simulink model "All_64_Coils"  (R2021b) (continued)
== system All_64_Coils/Coil-29 ==
  x : Inport
  y : Inport  Port=2
  z : Inport  Port=3
  Current : Inport  Port=4
  Add{,1..4} (5 alike) : Sum
  Display{,1..3} (4 alike) : Display
  Inner_5_coils : Subsystem
  Outer_5_Coils : Subsystem
  Scope : Scope  NumInputPorts=4
  Scope1 : Scope
  wires:
  Outer_5_Coils/3(Output2) -> Add2/2
  Inner_5_coils/2(Output1) -> Add1/1
  Outer_5_Coils/4(Output3) -> Add3/2
  z/1 -> Outer_5_Coils/3(Input2), Inner_5_coils/3(Input2)
  Add/1 -> Scope/1, Display/1  "Fxx"
  y/1 -> Outer_5_Coils/2(Input1), Inner_5_coils/2(Input1)
  Inner_5_coils/4(Output3) -> Add3/1
  Inner_5_coils/1(Output) -> Add/1
  Add3/1 -> Scope/4, Display3/1, Add4/2  "Fyz"
  Add1/1 -> Scope/2, Display1/1, Add4/1  "Fxz"
  Outer_5_Coils/1(Output) -> Add/2
  x/1 -> Outer_5_Coils/1(Input), Inner_5_coils/1(Input)
  Add2/1 -> Scope/3, Display2/1  "Fyy"
  Outer_5_Coils/2(Output1) -> Add1/2
  Current/1 -> Outer_5_Coils/4(Input3), Inner_5_coils/4(Input3)
  Inner_5_coils/3(Output2) -> Add2/1
  Add4/1 -> Scope1/1  "Total_Force"

== system All_64_Coils/Coil-3 ==
  x : Inport
  y : Inport  Port=2
  z : Inport  Port=3
  Current : Inport  Port=4
  Add{,1..4} (5 alike) : Sum
  Display{,1..3} (4 alike) : Display
  Inner_5_coils : Subsystem
  Outer_5_Coils : Subsystem
  Scope : Scope  NumInputPorts=4
  Scope1 : Scope
  wires:
  Outer_5_Coils/3(Output2) -> Add2/2
  Inner_5_coils/2(Output1) -> Add1/1
  Outer_5_Coils/4(Output3) -> Add3/2
  z/1 -> Outer_5_Coils/3(Input2), Inner_5_coils/3(Input2)
  Add/1 -> Scope/1, Display/1  "Fxx"
  y/1 -> Outer_5_Coils/2(Input1), Inner_5_coils/2(Input1)
  Inner_5_coils/4(Output3) -> Add3/1
  Inner_5_coils/1(Output) -> Add/1
  Add3/1 -> Scope/4, Display3/1, Add4/2  "Fyz"
  Add1/1 -> Scope/2, Display1/1, Add4/1  "Fxz"
  Outer_5_Coils/1(Output) -> Add/2
  x/1 -> Outer_5_Coils/1(Input), Inner_5_coils/1(Input)
  Add2/1 -> Scope/3, Display2/1  "Fyy"
  Outer_5_Coils/2(Output1) -> Add1/2
  Current/1 -> Outer_5_Coils/4(Input3), Inner_5_coils/4(Input3)
  Inner_5_coils/3(Output2) -> Add2/1
  Add4/1 -> Scope1/1  "Total_Force"

== system All_64_Coils/Coil-30 ==
  x : Inport
  y : Inport  Port=2
  z : Inport  Port=3
  Current : Inport  Port=4
  Add{,1..4} (5 alike) : Sum
  Display{,1..3} (4 alike) : Display
  Inner_5_coils : Subsystem
  Outer_5_Coils : Subsystem
  Scope : Scope  NumInputPorts=4
  Scope1 : Scope
  wires:
  Outer_5_Coils/3(Output2) -> Add2/2
  Inner_5_coils/2(Output1) -> Add1/1
  Outer_5_Coils/4(Output3) -> Add3/2
  z/1 -> Outer_5_Coils/3(Input2), Inner_5_coils/3(Input2)
  Add/1 -> Scope/1, Display/1  "Fxx"
  y/1 -> Outer_5_Coils/2(Input1), Inner_5_coils/2(Input1)
  Inner_5_coils/4(Output3) -> Add3/1
  Inner_5_coils/1(Output) -> Add/1
  Add3/1 -> Scope/4, Display3/1, Add4/2  "Fyz"
  Add1/1 -> Scope/2, Display1/1, Add4/1  "Fxz"
  Outer_5_Coils/1(Output) -> Add/2
  x/1 -> Outer_5_Coils/1(Input), Inner_5_coils/1(Input)
  Add2/1 -> Scope/3, Display2/1  "Fyy"
  Outer_5_Coils/2(Output1) -> Add1/2
  Current/1 -> Outer_5_Coils/4(Input3), Inner_5_coils/4(Input3)
  Inner_5_coils/3(Output2) -> Add2/1
  Add4/1 -> Scope1/1  "Total_Force"

== system All_64_Coils/Coil-31 ==
  x : Inport
  y : Inport  Port=2
  z : Inport  Port=3
  Current : Inport  Port=4
  Add{,1..4} (5 alike) : Sum
  Display{,1..3} (4 alike) : Display
  Inner_5_coils : Subsystem
  Outer_5_Coils : Subsystem
  Scope : Scope  NumInputPorts=4
  Scope1 : Scope
  wires:
  Add4/1 -> Scope1/1  "Total_Force"
  Inner_5_coils/3(Output2) -> Add2/1
  Current/1 -> Inner_5_coils/4(Input3), Outer_5_Coils/4(Input3)
  Outer_5_Coils/2(Output1) -> Add1/2
  Add2/1 -> Display2/1, Scope/3  "Fyy"
  x/1 -> Inner_5_coils/1(Input), Outer_5_Coils/1(Input)
  Outer_5_Coils/1(Output) -> Add/2
  Add1/1 -> Add4/1, Display1/1, Scope/2  "Fxz"
  Add3/1 -> Add4/2, Display3/1, Scope/4  "Fyz"
  Inner_5_coils/1(Output) -> Add/1
  Inner_5_coils/4(Output3) -> Add3/1
  y/1 -> Inner_5_coils/2(Input1), Outer_5_Coils/2(Input1)
  Add/1 -> Display/1, Scope/1  "Fxx"
  z/1 -> Inner_5_coils/3(Input2), Outer_5_Coils/3(Input2)
  Outer_5_Coils/4(Output3) -> Add3/2
  Inner_5_coils/2(Output1) -> Add1/1
  Outer_5_Coils/3(Output2) -> Add2/2

== system All_64_Coils/Coil-32 ==
  x : Inport
  y : Inport  Port=2
  z : Inport  Port=3
  Current : Inport  Port=4
  Add{,1..4} (5 alike) : Sum
  Display{,1..3} (4 alike) : Display
  Inner_5_coils : Subsystem
  Outer_5_Coils : Subsystem
  Scope : Scope  NumInputPorts=4
  Scope1 : Scope
  wires:
  Add4/1 -> Scope1/1  "Total_Force"
  Inner_5_coils/3(Output2) -> Add2/1
  Current/1 -> Inner_5_coils/4(Input3), Outer_5_Coils/4(Input3)
  Outer_5_Coils/2(Output1) -> Add1/2
  Add2/1 -> Display2/1, Scope/3  "Fyy"
  x/1 -> Inner_5_coils/1(Input), Outer_5_Coils/1(Input)
  Outer_5_Coils/1(Output) -> Add/2
  Add1/1 -> Add4/1, Display1/1, Scope/2  "Fxz"
  Add3/1 -> Add4/2, Display3/1, Scope/4  "Fyz"
  Inner_5_coils/1(Output) -> Add/1
  Inner_5_coils/4(Output3) -> Add3/1
  y/1 -> Inner_5_coils/2(Input1), Outer_5_Coils/2(Input1)
  Add/1 -> Display/1, Scope/1  "Fxx"
  z/1 -> Inner_5_coils/3(Input2), Outer_5_Coils/3(Input2)
  Outer_5_Coils/4(Output3) -> Add3/2
  Inner_5_coils/2(Output1) -> Add1/1
  Outer_5_Coils/3(Output2) -> Add2/2

== system All_64_Coils/Coil-33 ==
  x : Inport
  y : Inport  Port=2
  z : Inport  Port=3
  Current : Inport  Port=4
  Add{,1..4} (5 alike) : Sum
  Display{,1..3} (4 alike) : Display
  Inner_5_coils : Subsystem
  Outer_5_Coils : Subsystem
  Scope : Scope  NumInputPorts=4
  Scope1 : Scope
  wires:
  Outer_5_Coils/3(Output2) -> Add2/2
  Inner_5_coils/2(Output1) -> Add1/1
  Outer_5_Coils/4(Output3) -> Add3/2
  z/1 -> Outer_5_Coils/3(Input2), Inner_5_coils/3(Input2)
  Add/1 -> Scope/1, Display/1  "Fxx"
  y/1 -> Outer_5_Coils/2(Input1), Inner_5_coils/2(Input1)
  Inner_5_coils/4(Output3) -> Add3/1
  Inner_5_coils/1(Output) -> Add/1
  Add3/1 -> Scope/4, Display3/1, Add4/2  "Fyz"
  Add1/1 -> Scope/2, Display1/1, Add4/1  "Fxz"
  Outer_5_Coils/1(Output) -> Add/2
  x/1 -> Outer_5_Coils/1(Input), Inner_5_coils/1(Input)
  Add2/1 -> Scope/3, Display2/1  "Fyy"
  Outer_5_Coils/2(Output1) -> Add1/2
  Current/1 -> Outer_5_Coils/4(Input3), Inner_5_coils/4(Input3)
  Inner_5_coils/3(Output2) -> Add2/1
  Add4/1 -> Scope1/1  "Total_Force"

== system All_64_Coils/Coil-34 ==
  x : Inport
  y : Inport  Port=2
  z : Inport  Port=3
  Current : Inport  Port=4
  Add{,1..4} (5 alike) : Sum
  Display{,1..3} (4 alike) : Display
  Inner_5_coils : Subsystem
  Outer_5_Coils : Subsystem
  Scope : Scope  NumInputPorts=4
  Scope1 : Scope
  wires:
  Outer_5_Coils/3(Output2) -> Add2/2
  Inner_5_coils/2(Output1) -> Add1/1
  Outer_5_Coils/4(Output3) -> Add3/2
  z/1 -> Outer_5_Coils/3(Input2), Inner_5_coils/3(Input2)
  Add/1 -> Scope/1, Display/1  "Fxx"
  y/1 -> Outer_5_Coils/2(Input1), Inner_5_coils/2(Input1)
  Inner_5_coils/4(Output3) -> Add3/1
  Inner_5_coils/1(Output) -> Add/1
  Add3/1 -> Scope/4, Display3/1, Add4/2  "Fyz"
  Add1/1 -> Scope/2, Display1/1, Add4/1  "Fxz"
  Outer_5_Coils/1(Output) -> Add/2
  x/1 -> Outer_5_Coils/1(Input), Inner_5_coils/1(Input)
  Add2/1 -> Scope/3, Display2/1  "Fyy"
  Outer_5_Coils/2(Output1) -> Add1/2
  Current/1 -> Outer_5_Coils/4(Input3), Inner_5_coils/4(Input3)
  Inner_5_coils/3(Output2) -> Add2/1
  Add4/1 -> Scope1/1  "Total_Force"

== system All_64_Coils/Coil-35 ==
  x : Inport
  y : Inport  Port=2
  z : Inport  Port=3
  Current : Inport  Port=4
  Add{,1..4} (5 alike) : Sum
  Display{,1..3} (4 alike) : Display
  Inner_5_coils : Subsystem
  Outer_5_Coils : Subsystem
  Scope : Scope  NumInputPorts=4
  Scope1 : Scope
  wires:
  Outer_5_Coils/3(Output2) -> Add2/2
  Inner_5_coils/2(Output1) -> Add1/1
  Outer_5_Coils/4(Output3) -> Add3/2
  z/1 -> Outer_5_Coils/3(Input2), Inner_5_coils/3(Input2)
  Add/1 -> Scope/1, Display/1  "Fxx"
  y/1 -> Outer_5_Coils/2(Input1), Inner_5_coils/2(Input1)
  Inner_5_coils/4(Output3) -> Add3/1
  Inner_5_coils/1(Output) -> Add/1
  Add3/1 -> Scope/4, Display3/1, Add4/2  "Fyz"
  Add1/1 -> Scope/2, Display1/1, Add4/1  "Fxz"
  Outer_5_Coils/1(Output) -> Add/2
  x/1 -> Outer_5_Coils/1(Input), Inner_5_coils/1(Input)
  Add2/1 -> Scope/3, Display2/1  "Fyy"
  Outer_5_Coils/2(Output1) -> Add1/2
  Current/1 -> Outer_5_Coils/4(Input3), Inner_5_coils/4(Input3)
  Inner_5_coils/3(Output2) -> Add2/1
  Add4/1 -> Scope1/1  "Total_Force"

== system All_64_Coils/Coil-36 ==
  x : Inport
  y : Inport  Port=2
  z : Inport  Port=3
  Current : Inport  Port=4
  Add{,1..4} (5 alike) : Sum
  Display{,1..3} (4 alike) : Display
  Inner_5_coils : Subsystem
  Outer_5_Coils : Subsystem
  Scope : Scope  NumInputPorts=4
  Scope1 : Scope
  wires:
  Add4/1 -> Scope1/1  "Total_Force"
  Inner_5_coils/3(Output2) -> Add2/1
  Current/1 -> Inner_5_coils/4(Input3), Outer_5_Coils/4(Input3)
  Outer_5_Coils/2(Output1) -> Add1/2
  Add2/1 -> Display2/1, Scope/3  "Fyy"
  x/1 -> Inner_5_coils/1(Input), Outer_5_Coils/1(Input)
  Outer_5_Coils/1(Output) -> Add/2
  Add1/1 -> Add4/1, Display1/1, Scope/2  "Fxz"
  Add3/1 -> Add4/2, Display3/1, Scope/4  "Fyz"
  Inner_5_coils/1(Output) -> Add/1
  Inner_5_coils/4(Output3) -> Add3/1
  y/1 -> Inner_5_coils/2(Input1), Outer_5_Coils/2(Input1)
  Add/1 -> Display/1, Scope/1  "Fxx"
  z/1 -> Inner_5_coils/3(Input2), Outer_5_Coils/3(Input2)
  Outer_5_Coils/4(Output3) -> Add3/2
  Inner_5_coils/2(Output1) -> Add1/1
  Outer_5_Coils/3(Output2) -> Add2/2

== system All_64_Coils/Coil-37 ==
  x : Inport
  y : Inport  Port=2
  z : Inport  Port=3
  Current : Inport  Port=4
  Add{,1..4} (5 alike) : Sum
  Display{,1..3} (4 alike) : Display
  Inner_5_coils : Subsystem
  Outer_5_Coils : Subsystem
  Scope : Scope  NumInputPorts=4
  Scope1 : Scope
  wires:
  Outer_5_Coils/3(Output2) -> Add2/2
  Inner_5_coils/2(Output1) -> Add1/1
  Outer_5_Coils/4(Output3) -> Add3/2
  z/1 -> Outer_5_Coils/3(Input2), Inner_5_coils/3(Input2)
  Add/1 -> Scope/1, Display/1  "Fxx"
  y/1 -> Outer_5_Coils/2(Input1), Inner_5_coils/2(Input1)
  Inner_5_coils/4(Output3) -> Add3/1
  Inner_5_coils/1(Output) -> Add/1
  Add3/1 -> Scope/4, Display3/1, Add4/2  "Fyz"
  Add1/1 -> Scope/2, Display1/1, Add4/1  "Fxz"
  Outer_5_Coils/1(Output) -> Add/2
  x/1 -> Outer_5_Coils/1(Input), Inner_5_coils/1(Input)
  Add2/1 -> Scope/3, Display2/1  "Fyy"
  Outer_5_Coils/2(Output1) -> Add1/2
  Current/1 -> Outer_5_Coils/4(Input3), Inner_5_coils/4(Input3)
  Inner_5_coils/3(Output2) -> Add2/1
  Add4/1 -> Scope1/1  "Total_Force"

== system All_64_Coils/Coil-38 ==
  x : Inport
  y : Inport  Port=2
  z : Inport  Port=3
  Current : Inport  Port=4
  Add{,1..4} (5 alike) : Sum
  Display{,1..3} (4 alike) : Display
  Inner_5_coils : Subsystem
  Outer_5_Coils : Subsystem
  Scope : Scope  NumInputPorts=4
  Scope1 : Scope
  wires:
  Add4/1 -> Scope1/1  "Total_Force"
  Inner_5_coils/3(Output2) -> Add2/1
  Current/1 -> Inner_5_coils/4(Input3), Outer_5_Coils/4(Input3)
  Outer_5_Coils/2(Output1) -> Add1/2
  Add2/1 -> Display2/1, Scope/3  "Fyy"
  x/1 -> Inner_5_coils/1(Input), Outer_5_Coils/1(Input)
  Outer_5_Coils/1(Output) -> Add/2
  Add1/1 -> Add4/1, Display1/1, Scope/2  "Fxz"
  Add3/1 -> Add4/2, Display3/1, Scope/4  "Fyz"
  Inner_5_coils/1(Output) -> Add/1
  Inner_5_coils/4(Output3) -> Add3/1
  y/1 -> Inner_5_coils/2(Input1), Outer_5_Coils/2(Input1)
  Add/1 -> Display/1, Scope/1  "Fxx"
  z/1 -> Inner_5_coils/3(Input2), Outer_5_Coils/3(Input2)
  Outer_5_Coils/4(Output3) -> Add3/2
  Inner_5_coils/2(Output1) -> Add1/1
  Outer_5_Coils/3(Output2) -> Add2/2

== system All_64_Coils/Coil-39 ==
  x : Inport
  y : Inport  Port=2
  z : Inport  Port=3
  Current : Inport  Port=4
  Add{,1..4} (5 alike) : Sum
  Display{,1..3} (4 alike) : Display
  Inner_5_coils : Subsystem
  Outer_5_Coils : Subsystem
  Scope : Scope  NumInputPorts=4
  Scope1 : Scope
  wires:
  Add4/1 -> Scope1/1  "Total_Force"
  Inner_5_coils/3(Output2) -> Add2/1
  Current/1 -> Inner_5_coils/4(Input3), Outer_5_Coils/4(Input3)
  Outer_5_Coils/2(Output1) -> Add1/2
  Add2/1 -> Display2/1, Scope/3  "Fyy"
  x/1 -> Inner_5_coils/1(Input), Outer_5_Coils/1(Input)
  Outer_5_Coils/1(Output) -> Add/2
  Add1/1 -> Add4/1, Display1/1, Scope/2  "Fxz"
  Add3/1 -> Add4/2, Display3/1, Scope/4  "Fyz"
  Inner_5_coils/1(Output) -> Add/1
  Inner_5_coils/4(Output3) -> Add3/1
  y/1 -> Inner_5_coils/2(Input1), Outer_5_Coils/2(Input1)
  Add/1 -> Display/1, Scope/1  "Fxx"
  z/1 -> Inner_5_coils/3(Input2), Outer_5_Coils/3(Input2)
  Outer_5_Coils/4(Output3) -> Add3/2
  Inner_5_coils/2(Output1) -> Add1/1
  Outer_5_Coils/3(Output2) -> Add2/2

== system All_64_Coils/Coil-4 ==
  x : Inport
  y : Inport  Port=2
  z : Inport  Port=3
  Current : Inport  Port=4
  Add{,1..4} (5 alike) : Sum
  Display{,1..3} (4 alike) : Display
  Inner_5_coils : Subsystem
  Outer_5_Coils : Subsystem
  Scope : Scope  NumInputPorts=4
  Scope1 : Scope
  wires:
  Add4/1 -> Scope1/1  "Total_Force"
  Inner_5_coils/3(Output2) -> Add2/1
  Current/1 -> Inner_5_coils/4(Input3), Outer_5_Coils/4(Input3)
  Outer_5_Coils/2(Output1) -> Add1/2
  Add2/1 -> Display2/1, Scope/3  "Fyy"
  x/1 -> Inner_5_coils/1(Input), Outer_5_Coils/1(Input)
  Outer_5_Coils/1(Output) -> Add/2
  Add1/1 -> Add4/1, Display1/1, Scope/2  "Fxz"
  Add3/1 -> Add4/2, Display3/1, Scope/4  "Fyz"
  Inner_5_coils/1(Output) -> Add/1
  Inner_5_coils/4(Output3) -> Add3/1
  y/1 -> Inner_5_coils/2(Input1), Outer_5_Coils/2(Input1)
  Add/1 -> Display/1, Scope/1  "Fxx"
  z/1 -> Inner_5_coils/3(Input2), Outer_5_Coils/3(Input2)
  Outer_5_Coils/4(Output3) -> Add3/2
  Inner_5_coils/2(Output1) -> Add1/1
  Outer_5_Coils/3(Output2) -> Add2/2

== system All_64_Coils/Coil-40 ==
  x : Inport
  y : Inport  Port=2
  z : Inport  Port=3
  Current : Inport  Port=4
  Add{,1..4} (5 alike) : Sum
  Display{,1..3} (4 alike) : Display
  Inner_5_coils : Subsystem
  Outer_5_Coils : Subsystem
  Scope : Scope  NumInputPorts=4
  Scope1 : Scope
  wires:
  Outer_5_Coils/3(Output2) -> Add2/2
  Inner_5_coils/2(Output1) -> Add1/1
  Outer_5_Coils/4(Output3) -> Add3/2
  z/1 -> Outer_5_Coils/3(Input2), Inner_5_coils/3(Input2)
  Add/1 -> Scope/1, Display/1  "Fxx"
  y/1 -> Outer_5_Coils/2(Input1), Inner_5_coils/2(Input1)
  Inner_5_coils/4(Output3) -> Add3/1
  Inner_5_coils/1(Output) -> Add/1
  Add3/1 -> Scope/4, Display3/1, Add4/2  "Fyz"
  Add1/1 -> Scope/2, Display1/1, Add4/1  "Fxz"
  Outer_5_Coils/1(Output) -> Add/2
  x/1 -> Outer_5_Coils/1(Input), Inner_5_coils/1(Input)
  Add2/1 -> Scope/3, Display2/1  "Fyy"
  Outer_5_Coils/2(Output1) -> Add1/2
  Current/1 -> Outer_5_Coils/4(Input3), Inner_5_coils/4(Input3)
  Inner_5_coils/3(Output2) -> Add2/1
  Add4/1 -> Scope1/1  "Total_Force"

== system All_64_Coils/Coil-41 ==
  x : Inport
  y : Inport  Port=2
  z : Inport  Port=3
  Current : Inport  Port=4
  Add{,1..4} (5 alike) : Sum
  Display{,1..3} (4 alike) : Display
  Inner_5_coils : Subsystem
  Outer_5_Coils : Subsystem
  Scope : Scope  NumInputPorts=4
  Scope1 : Scope
  wires:
  Add4/1 -> Scope1/1  "Total_Force"
  Inner_5_coils/3(Output2) -> Add2/1
  Current/1 -> Inner_5_coils/4(Input3), Outer_5_Coils/4(Input3)
  Outer_5_Coils/2(Output1) -> Add1/2
  Add2/1 -> Display2/1, Scope/3  "Fyy"
  x/1 -> Inner_5_coils/1(Input), Outer_5_Coils/1(Input)
  Outer_5_Coils/1(Output) -> Add/2
  Add1/1 -> Add4/1, Display1/1, Scope/2  "Fxz"
  Add3/1 -> Add4/2, Display3/1, Scope/4  "Fyz"
  Inner_5_coils/1(Output) -> Add/1
  Inner_5_coils/4(Output3) -> Add3/1
  y/1 -> Inner_5_coils/2(Input1), Outer_5_Coils/2(Input1)
  Add/1 -> Display/1, Scope/1  "Fxx"
  z/1 -> Inner_5_coils/3(Input2), Outer_5_Coils/3(Input2)
  Outer_5_Coils/4(Output3) -> Add3/2
  Inner_5_coils/2(Output1) -> Add1/1
  Outer_5_Coils/3(Output2) -> Add2/2

== system All_64_Coils/Coil-42 ==
  x : Inport
  y : Inport  Port=2
  z : Inport  Port=3
  Current : Inport  Port=4
  Add{,1..4} (5 alike) : Sum
  Display{,1..3} (4 alike) : Display
  Inner_5_coils : Subsystem
  Outer_5_Coils : Subsystem
  Scope : Scope  NumInputPorts=4
  Scope1 : Scope
  wires:
  Add4/1 -> Scope1/1  "Total_Force"
  Inner_5_coils/3(Output2) -> Add2/1
  Current/1 -> Inner_5_coils/4(Input3), Outer_5_Coils/4(Input3)
  Outer_5_Coils/2(Output1) -> Add1/2
  Add2/1 -> Display2/1, Scope/3  "Fyy"
  x/1 -> Inner_5_coils/1(Input), Outer_5_Coils/1(Input)
  Outer_5_Coils/1(Output) -> Add/2
  Add1/1 -> Add4/1, Display1/1, Scope/2  "Fxz"
  Add3/1 -> Add4/2, Display3/1, Scope/4  "Fyz"
  Inner_5_coils/1(Output) -> Add/1
  Inner_5_coils/4(Output3) -> Add3/1
  y/1 -> Inner_5_coils/2(Input1), Outer_5_Coils/2(Input1)
  Add/1 -> Display/1, Scope/1  "Fxx"
  z/1 -> Inner_5_coils/3(Input2), Outer_5_Coils/3(Input2)
  Outer_5_Coils/4(Output3) -> Add3/2
  Inner_5_coils/2(Output1) -> Add1/1
  Outer_5_Coils/3(Output2) -> Add2/2

== system All_64_Coils/Coil-43 ==
  x : Inport
  y : Inport  Port=2
  z : Inport  Port=3
  Current : Inport  Port=4
  Add{,1..4} (5 alike) : Sum
  Display{,1..3} (4 alike) : Display
  Inner_5_coils : Subsystem
  Outer_5_Coils : Subsystem
  Scope : Scope  NumInputPorts=4
  Scope1 : Scope
  wires:
  Add4/1 -> Scope1/1  "Total_Force"
  Inner_5_coils/3(Output2) -> Add2/1
  Current/1 -> Inner_5_coils/4(Input3), Outer_5_Coils/4(Input3)
  Outer_5_Coils/2(Output1) -> Add1/2
  Add2/1 -> Display2/1, Scope/3  "Fyy"
  x/1 -> Inner_5_coils/1(Input), Outer_5_Coils/1(Input)
  Outer_5_Coils/1(Output) -> Add/2
  Add1/1 -> Add4/1, Display1/1, Scope/2  "Fxz"
  Add3/1 -> Add4/2, Display3/1, Scope/4  "Fyz"
  Inner_5_coils/1(Output) -> Add/1
  Inner_5_coils/4(Output3) -> Add3/1
  y/1 -> Inner_5_coils/2(Input1), Outer_5_Coils/2(Input1)
  Add/1 -> Display/1, Scope/1  "Fxx"
  z/1 -> Inner_5_coils/3(Input2), Outer_5_Coils/3(Input2)
  Outer_5_Coils/4(Output3) -> Add3/2
  Inner_5_coils/2(Output1) -> Add1/1
  Outer_5_Coils/3(Output2) -> Add2/2

== system All_64_Coils/Coil-44 ==
  x : Inport
  y : Inport  Port=2
  z : Inport  Port=3
  Current : Inport  Port=4
  Add{,1..4} (5 alike) : Sum
  Display{,1..3} (4 alike) : Display
  Inner_5_coils : Subsystem
  Outer_5_Coils : Subsystem
  Scope : Scope  NumInputPorts=4
  Scope1 : Scope
  wires:
  Add4/1 -> Scope1/1  "Total_Force"
  Inner_5_coils/3(Output2) -> Add2/1
  Current/1 -> Inner_5_coils/4(Input3), Outer_5_Coils/4(Input3)
  Outer_5_Coils/2(Output1) -> Add1/2
  Add2/1 -> Display2/1, Scope/3  "Fyy"
  x/1 -> Inner_5_coils/1(Input), Outer_5_Coils/1(Input)
  Outer_5_Coils/1(Output) -> Add/2
  Add1/1 -> Add4/1, Display1/1, Scope/2  "Fxz"
  Add3/1 -> Add4/2, Display3/1, Scope/4  "Fyz"
  Inner_5_coils/1(Output) -> Add/1
  Inner_5_coils/4(Output3) -> Add3/1
  y/1 -> Inner_5_coils/2(Input1), Outer_5_Coils/2(Input1)
  Add/1 -> Display/1, Scope/1  "Fxx"
  z/1 -> Inner_5_coils/3(Input2), Outer_5_Coils/3(Input2)
  Outer_5_Coils/4(Output3) -> Add3/2
  Inner_5_coils/2(Output1) -> Add1/1
  Outer_5_Coils/3(Output2) -> Add2/2

== system All_64_Coils/Coil-45 ==
  x : Inport
  y : Inport  Port=2
  z : Inport  Port=3
  Current : Inport  Port=4
  Add{,1..4} (5 alike) : Sum
  Display{,1..3} (4 alike) : Display
  Inner_5_coils : Subsystem
  Outer_5_Coils : Subsystem
  Scope : Scope  NumInputPorts=4
  Scope1 : Scope
  wires:
  Outer_5_Coils/3(Output2) -> Add2/2
  Inner_5_coils/2(Output1) -> Add1/1
  Outer_5_Coils/4(Output3) -> Add3/2
  z/1 -> Outer_5_Coils/3(Input2), Inner_5_coils/3(Input2)
  Add/1 -> Scope/1, Display/1  "Fxx"
  y/1 -> Outer_5_Coils/2(Input1), Inner_5_coils/2(Input1)
  Inner_5_coils/4(Output3) -> Add3/1
  Inner_5_coils/1(Output) -> Add/1
  Add3/1 -> Scope/4, Display3/1, Add4/2  "Fyz"
  Add1/1 -> Scope/2, Display1/1, Add4/1  "Fxz"
  Outer_5_Coils/1(Output) -> Add/2
  x/1 -> Outer_5_Coils/1(Input), Inner_5_coils/1(Input)
  Add2/1 -> Scope/3, Display2/1  "Fyy"
  Outer_5_Coils/2(Output1) -> Add1/2
  Current/1 -> Outer_5_Coils/4(Input3), Inner_5_coils/4(Input3)
  Inner_5_coils/3(Output2) -> Add2/1
  Add4/1 -> Scope1/1  "Total_Force"

== system All_64_Coils/Coil-46 ==
  x : Inport
  y : Inport  Port=2
  z : Inport  Port=3
  Current : Inport  Port=4
  Add{,1..4} (5 alike) : Sum
  Display{,1..3} (4 alike) : Display
  Inner_5_coils : Subsystem
  Outer_5_Coils : Subsystem
  Scope : Scope  NumInputPorts=4
  Scope1 : Scope
  wires:
  Add4/1 -> Scope1/1  "Total_Force"
  Inner_5_coils/3(Output2) -> Add2/1
  Current/1 -> Inner_5_coils/4(Input3), Outer_5_Coils/4(Input3)
  Outer_5_Coils/2(Output1) -> Add1/2
  Add2/1 -> Display2/1, Scope/3  "Fyy"
  x/1 -> Inner_5_coils/1(Input), Outer_5_Coils/1(Input)
  Outer_5_Coils/1(Output) -> Add/2
  Add1/1 -> Add4/1, Display1/1, Scope/2  "Fxz"
  Add3/1 -> Add4/2, Display3/1, Scope/4  "Fyz"
  Inner_5_coils/1(Output) -> Add/1
  Inner_5_coils/4(Output3) -> Add3/1
  y/1 -> Inner_5_coils/2(Input1), Outer_5_Coils/2(Input1)
  Add/1 -> Display/1, Scope/1  "Fxx"
  z/1 -> Inner_5_coils/3(Input2), Outer_5_Coils/3(Input2)
  Outer_5_Coils/4(Output3) -> Add3/2
  Inner_5_coils/2(Output1) -> Add1/1
  Outer_5_Coils/3(Output2) -> Add2/2

== system All_64_Coils/Coil-47 ==
  x : Inport
  y : Inport  Port=2
  z : Inport  Port=3
  Current : Inport  Port=4
  Add{,1..4} (5 alike) : Sum
  Display{,1..3} (4 alike) : Display
  Inner_5_coils : Subsystem
  Outer_5_Coils : Subsystem
  Scope : Scope  NumInputPorts=4
  Scope1 : Scope
  wires:
  Outer_5_Coils/3(Output2) -> Add2/2
  Inner_5_coils/2(Output1) -> Add1/1
  Outer_5_Coils/4(Output3) -> Add3/2
  z/1 -> Outer_5_Coils/3(Input2), Inner_5_coils/3(Input2)
  Add/1 -> Scope/1, Display/1  "Fxx"
  y/1 -> Outer_5_Coils/2(Input1), Inner_5_coils/2(Input1)
  Inner_5_coils/4(Output3) -> Add3/1
  Inner_5_coils/1(Output) -> Add/1
  Add3/1 -> Scope/4, Display3/1, Add4/2  "Fyz"
  Add1/1 -> Scope/2, Display1/1, Add4/1  "Fxz"
  Outer_5_Coils/1(Output) -> Add/2
  x/1 -> Outer_5_Coils/1(Input), Inner_5_coils/1(Input)
  Add2/1 -> Scope/3, Display2/1  "Fyy"
  Outer_5_Coils/2(Output1) -> Add1/2
  Current/1 -> Outer_5_Coils/4(Input3), Inner_5_coils/4(Input3)
  Inner_5_coils/3(Output2) -> Add2/1
  Add4/1 -> Scope1/1  "Total_Force"

== system All_64_Coils/Coil-48 ==
  x : Inport
  y : Inport  Port=2
  z : Inport  Port=3
  Current : Inport  Port=4
  Add{,1..4} (5 alike) : Sum
  Display{,1..3} (4 alike) : Display
  Inner_5_coils : Subsystem
  Outer_5_Coils : Subsystem
  Scope : Scope  NumInputPorts=4
  Scope1 : Scope
  wires:
  Outer_5_Coils/3(Output2) -> Add2/2
  Inner_5_coils/2(Output1) -> Add1/1
  Outer_5_Coils/4(Output3) -> Add3/2
  z/1 -> Outer_5_Coils/3(Input2), Inner_5_coils/3(Input2)
  Add/1 -> Scope/1, Display/1  "Fxx"
  y/1 -> Outer_5_Coils/2(Input1), Inner_5_coils/2(Input1)
  Inner_5_coils/4(Output3) -> Add3/1
  Inner_5_coils/1(Output) -> Add/1
  Add3/1 -> Scope/4, Display3/1, Add4/2  "Fyz"
  Add1/1 -> Scope/2, Display1/1, Add4/1  "Fxz"
  Outer_5_Coils/1(Output) -> Add/2
  x/1 -> Outer_5_Coils/1(Input), Inner_5_coils/1(Input)
  Add2/1 -> Scope/3, Display2/1  "Fyy"
  Outer_5_Coils/2(Output1) -> Add1/2
  Current/1 -> Outer_5_Coils/4(Input3), Inner_5_coils/4(Input3)
  Inner_5_coils/3(Output2) -> Add2/1
  Add4/1 -> Scope1/1  "Total_Force"

== system All_64_Coils/Coil-49 ==
  x : Inport
  y : Inport  Port=2
  z : Inport  Port=3
  Current : Inport  Port=4
  Add{,1..4} (5 alike) : Sum
  Display{,1..3} (4 alike) : Display
  Inner_5_coils : Subsystem
  Outer_5_Coils : Subsystem
  Scope : Scope  NumInputPorts=4
  Scope1 : Scope
  wires:
  Add4/1 -> Scope1/1  "Total_Force"
  Inner_5_coils/3(Output2) -> Add2/1
  Current/1 -> Inner_5_coils/4(Input3), Outer_5_Coils/4(Input3)
  Outer_5_Coils/2(Output1) -> Add1/2
  Add2/1 -> Display2/1, Scope/3  "Fyy"
  x/1 -> Inner_5_coils/1(Input), Outer_5_Coils/1(Input)
  Outer_5_Coils/1(Output) -> Add/2
  Add1/1 -> Add4/1, Display1/1, Scope/2  "Fxz"
  Add3/1 -> Add4/2, Display3/1, Scope/4  "Fyz"
  Inner_5_coils/1(Output) -> Add/1
  Inner_5_coils/4(Output3) -> Add3/1
  y/1 -> Inner_5_coils/2(Input1), Outer_5_Coils/2(Input1)
  Add/1 -> Display/1, Scope/1  "Fxx"
  z/1 -> Inner_5_coils/3(Input2), Outer_5_Coils/3(Input2)
  Outer_5_Coils/4(Output3) -> Add3/2
  Inner_5_coils/2(Output1) -> Add1/1
  Outer_5_Coils/3(Output2) -> Add2/2

== system All_64_Coils/Coil-5 ==
  x : Inport
  y : Inport  Port=2
  z : Inport  Port=3
  Current : Inport  Port=4
  Add{,1..4} (5 alike) : Sum
  Display{,1..3} (4 alike) : Display
  Inner_5_coils : Subsystem
  Outer_5_Coils : Subsystem
  Scope : Scope  NumInputPorts=4
  Scope1 : Scope
  wires:
  Outer_5_Coils/3(Output2) -> Add2/2
  Inner_5_coils/2(Output1) -> Add1/1
  Outer_5_Coils/4(Output3) -> Add3/2
  z/1 -> Outer_5_Coils/3(Input2), Inner_5_coils/3(Input2)
  Add/1 -> Scope/1, Display/1  "Fxx"
  y/1 -> Outer_5_Coils/2(Input1), Inner_5_coils/2(Input1)
  Inner_5_coils/4(Output3) -> Add3/1
  Inner_5_coils/1(Output) -> Add/1
  Add3/1 -> Scope/4, Display3/1, Add4/2  "Fyz"
  Add1/1 -> Scope/2, Display1/1, Add4/1  "Fxz"
  Outer_5_Coils/1(Output) -> Add/2
  x/1 -> Outer_5_Coils/1(Input), Inner_5_coils/1(Input)
  Add2/1 -> Scope/3, Display2/1  "Fyy"
  Outer_5_Coils/2(Output1) -> Add1/2
  Current/1 -> Outer_5_Coils/4(Input3), Inner_5_coils/4(Input3)
  Inner_5_coils/3(Output2) -> Add2/1
  Add4/1 -> Scope1/1  "Total_Force"

== system All_64_Coils/Coil-50 ==
  x : Inport
  y : Inport  Port=2
  z : Inport  Port=3
  Current : Inport  Port=4
  Add{,1..4} (5 alike) : Sum
  Display{,1..3} (4 alike) : Display
  Inner_5_coils : Subsystem
  Outer_5_Coils : Subsystem
  Scope : Scope  NumInputPorts=4
  Scope1 : Scope
  wires:
  Outer_5_Coils/3(Output2) -> Add2/2
  Inner_5_coils/2(Output1) -> Add1/1
  Outer_5_Coils/4(Output3) -> Add3/2
  z/1 -> Outer_5_Coils/3(Input2), Inner_5_coils/3(Input2)
  Add/1 -> Scope/1, Display/1  "Fxx"
  y/1 -> Outer_5_Coils/2(Input1), Inner_5_coils/2(Input1)
  Inner_5_coils/4(Output3) -> Add3/1
  Inner_5_coils/1(Output) -> Add/1
  Add3/1 -> Scope/4, Display3/1, Add4/2  "Fyz"
  Add1/1 -> Scope/2, Display1/1, Add4/1  "Fxz"
  Outer_5_Coils/1(Output) -> Add/2
  x/1 -> Outer_5_Coils/1(Input), Inner_5_coils/1(Input)
  Add2/1 -> Scope/3, Display2/1  "Fyy"
  Outer_5_Coils/2(Output1) -> Add1/2
  Current/1 -> Outer_5_Coils/4(Input3), Inner_5_coils/4(Input3)
  Inner_5_coils/3(Output2) -> Add2/1
  Add4/1 -> Scope1/1  "Total_Force"
